simulink model "wlan_phy_rx_pmd"  (Simulink 7.8)
settings: Solver=VariableStepDiscrete  SolverMode=SingleTasking  StartTime=0.0  StopTime=simtime  RelTol=1e-3
PostLoadFcn:
  | wlan_phy_rx_init
InitFcn:
  | wlan_phy_rx_init
StartFcn:
  | wlan_phy_rx_init
values from neighbouring files (symbols this model uses):
  barker_seq20 = 0.95 * circshift(barker_seq20, [0 4]) ./ max(abs(barker_seq20))    [wlan_phy_rx_init.m]
  bit_scrambler_lfsr_bytes = bi2de(reshape(repmat(scr, 1, 8), 8, 127)', 'left-msb')    [wlan_phy_rx_init.m]
  deinterleave_ROM = [deinterleave_ROM interleave_64QAM zeros(1, 512-length(interleave_64QAM))]    [wlan_phy_rx_init.m]
  longCorr_coef_i = [3*real(LTS_corr)]    [wlan_phy_rx_init.m]
  longCorr_coef_q = [3*imag(LTS_corr)]    [wlan_phy_rx_init.m]
  MAX_CP_LEN = 32    [wlan_phy_rx_init.m]
  MAX_NCBPS = 288    [wlan_phy_rx_init.m]
  MAX_NUM_BYTES = 4096    [wlan_phy_rx_init.m]
  MAX_NUM_SC = 64    [wlan_phy_rx_init.m]
  MAX_NUM_SYMS = 600    [wlan_phy_rx_init.m]
  n_bits_preFFT_sampBuff = ceil(log2(4*MAX_NUM_SC))    [wlan_phy_rx_init.m]
  NCBPS_16QAM = 192    [wlan_phy_rx_init.m]
  NCBPS_64QAM = 288    [wlan_phy_rx_init.m]
  NCBPS_BPSK = 48    [wlan_phy_rx_init.m]
  NCBPS_QPSK = 96    [wlan_phy_rx_init.m]
  NDBPS_16QAM12 = 96    [wlan_phy_rx_init.m]
  NDBPS_16QAM34 = 144    [wlan_phy_rx_init.m]
  NDBPS_64QAM23 = 192    [wlan_phy_rx_init.m]
  NDBPS_64QAM34 = 216    [wlan_phy_rx_init.m]
  NDBPS_BPSK12 = 24    [wlan_phy_rx_init.m]
  NDBPS_BPSK34 = 36    [wlan_phy_rx_init.m]
  NDBPS_QPSK12 = 48    [wlan_phy_rx_init.m]
  NDBPS_QPSK34 = 72    [wlan_phy_rx_init.m]
  paylod_vec_samp_time = 8    [wlan_phy_rx_init.m]
  PHY_MIN_PKT_LEN = 14    [wlan_phy_rx_init.m]
  scr_ind_rev = zeros(1,128)    [wlan_phy_rx_init.m]
undefined symbols (not defined in the file or in its folder): gcbh, xlBlockLoadCallback, xlGetNormalizedPeriod, xlipgetpartsetting
libraries: xbsIndex_r4 x3519, simulink x1, xbsEDKLib_r4 x1
(part 4 of 21)

== system wlan_phy_rx_pmd/CFO & Samp Buffer/CFO Correction ==
  note: DDS requires >1 cycle EN early to get setup
      | Use gloabl reset for this
      | Safe, since DDS output is ignored when
      | it's not being used, so bogus outputs
      | between packets is no problem
      |
      | DDS updates once per input sample
      | Since the FFT only consumes 64 samples
      | per OFDM symbol (ignoring the CP),
      | the DDS keeps running after each FFT
      | frame is loaded. This is fine, since the FFT is
      | configured for non-streaming (it ignores its
      | I/Q inputs while computing a transform)
  "I " : Inport
  "Q " : Inport  Port=2
  DDS Run : Inport  Port=3
  Valid : Inport  Port=4
  tlast : Inport  Port=5
  CFO Est : Inport  Port=6
  CFO Corr : Scope  NumInputPorts=8
      settings: TimeRange=35000  YMin=0~-0.4~0~-1~0~0~-0.4~0  YMax=1~0.4~1~2~1~1~0.4~1  SaveName=ScopeData3
  "Complex Multiplier 5.0 " : xbsIndex_r4/Complex Multiplier 5.0   hasatlast=off  hasatuser=on  atuserwidth=1  hasbtlast=off  hasbtuser=off  btuserwidth=1  multtype=Use_Mults  optimizegoal=Performance  flowcontrol=NonBlocking  outputwidth=16  roundmode=Truncate  hasctrltlast=off  hasctrltuser=off  ctrltuserwidth=1  outtlastbehv=Null  latencyconfig=Manual  minimumlatency=2  aclken=off  aresetn=off  trim_axipin_name=on  aportwidth=63  bportwidth=63  ip_name=Complex Multiplier  ip_version=5.0  dsptool_ready=true  ipcore_usecache=true  ipcore_useipmodelcache=true  ipcore_verbose=false  ipcore_latency_parameter='minimumlatency'  wrapper_available=true  port_translation_map={ 'aclken' => 'en', 'aresetn' => 'rst'}  structural_sim=false  block_type=cmpy_v5_0
  Constant1 : xbsIndex_r4/Constant  const=1  gui_display_data_type=Boolean  arith_type=Boolean  n_bits=16  bin_pt=14  preci_type=Single  exp_width=8  frac_width=24  explicit_period=off  period=8  equ=P=C  opselect=C  inp2=PCIN>>17  opr=+  inp1=P  carry=CIN  dbl_ovrd=off  block_type=constant
  Constant2 : xbsIndex_r4/Constant  const=1  gui_display_data_type=Fixed-point  arith_type=Signed (2's comp)  n_bits=16  bin_pt=15  preci_type=Single  exp_width=8  frac_width=24  explicit_period=on  period=1  equ=P=C  opselect=C  inp2=PCIN>>17  opr=+  inp1=P  carry=CIN  dbl_ovrd=off  block_type=constant
  Constant3 : xbsIndex_r4/Constant  const=0  gui_display_data_type=Fixed-point  arith_type=Signed (2's comp)  n_bits=16  bin_pt=15  preci_type=Single  exp_width=8  frac_width=24  explicit_period=on  period=1  equ=P=C  opselect=C  inp2=PCIN>>17  opr=+  inp1=P  carry=CIN  dbl_ovrd=off  block_type=constant
  Constant5 : xbsIndex_r4/Constant  const=1  gui_display_data_type=Boolean  arith_type=Boolean  n_bits=16  bin_pt=14  preci_type=Single  exp_width=8  frac_width=24  explicit_period=off  period=8  equ=P=C  opselect=C  inp2=PCIN>>17  opr=+  inp1=P  carry=CIN  dbl_ovrd=off  block_type=constant
  Convert1 : xbsIndex_r4/Convert  gui_display_data_type=Fixed-point  arith_type="Signed  (2's comp)"  n_bits=16  bin_pt=15  float_type=Single  exp_bits=8  fraction_bits=24  quantization=Truncate  overflow=Wrap  en=off  latency=0  dbl_ovrd=off  pipeline=off  block_type=convert
  Convert2 : xbsIndex_r4/Convert  gui_display_data_type=Fixed-point  arith_type="Signed  (2's comp)"  n_bits=16  bin_pt=15  float_type=Single  exp_bits=8  fraction_bits=24  quantization=Truncate  overflow=Wrap  en=off  latency=0  dbl_ovrd=off  pipeline=off  block_type=convert
  Convert3 : xbsIndex_r4/Convert  gui_display_data_type=Fixed-point  arith_type="Signed  (2's comp)"  n_bits=26  bin_pt=26  float_type=Single  exp_bits=8  fraction_bits=24  quantization=Truncate  overflow=Flag as error  en=off  latency=0  dbl_ovrd=off  pipeline=off  block_type=convert
  Convert4 : xbsIndex_r4/Convert  gui_display_data_type=Fixed-point  arith_type=Unsigned  n_bits=1  bin_pt=0  float_type=Single  exp_bits=8  fraction_bits=24  quantization=Truncate  overflow=Flag as error  en=off  latency=0  dbl_ovrd=off  pipeline=off  block_type=convert
  Convert5 : xbsIndex_r4/Convert  gui_display_data_type=Boolean  arith_type=Boolean  n_bits=1  bin_pt=0  float_type=Single  exp_bits=8  fraction_bits=24  quantization=Truncate  overflow=Flag as error  en=off  latency=0  dbl_ovrd=off  pipeline=off  block_type=convert
  DDS Compiler 4.0 : xbsIndex_r4/DDS Compiler 4.0   partspresent=Phase_Generator_and_SIN_COS_LUT  dds_clock_rate=160  channels=1  parameter_entry=Hardware_Parameters  spurious_free_dynamic_range=36  frequency_resolution=0.4  noise_shaping=None  phase_width=26  output_width=16  output_selection=Sine_and_Cosine  negative_sine=off  negative_cosine=off  amplitude_mode=Full_Range  memory_type=Auto  optimization_goal=Auto  dsp48_use=Minimal  latency_configuration=Auto  latency=6  has_phase_out=off  sclr_pin=off  clock_enable=on  rfd=off  rdy=on  channel_pin=off  explicit_period=off  period=1  phase_increment=Programmable  output_frequency1=0  output_frequency2=0  output_frequency3=0  output_frequency4=0  output_frequency5=0  output_frequency6=0  output_frequency7=0  output_frequency8=0  output_frequency9=0  output_frequency10=0  output_frequency11=0  output_frequency12=0  output_frequency13=0  output_frequency14=0  output_frequency15=0  output_frequency16=0  pinc1='0'  pinc2='0'  pinc3='0'  pinc4='0'  pinc5='0'  pinc6='0'  pinc7='0'  pinc8='0'  pinc9='0'  pinc10='0'  pinc11='0'  pinc12='0'  pinc13='0'  pinc14='0'  pinc15='0'  pinc16='0'  phase_offset=None  phase_offset_angles1=0  phase_offset_angles2=0  phase_offset_angles3=0  phase_offset_angles4=0  phase_offset_angles5=0  phase_offset_angles6=0  phase_offset_angles7=0  phase_offset_angles8=0  phase_offset_angles9=0  phase_offset_angles10=0  phase_offset_angles11=0  phase_offset_angles12=0  phase_offset_angles13=0  phase_offset_angles14=0  phase_offset_angles15=0  phase_offset_angles16=0  poff1='0'  poff2='0'  poff3='0'  poff4='0'  poff5='0'  poff6='0'  poff7='0'  poff8='0'  poff9='0'  poff10='0'  poff11='0'  poff12='0'  poff13='0'  poff14='0'  poff15='0'  poff16='0'  por_mode=false  gui_behaviour=Sysgen  ip_name=DDS Compiler  ip_version=4.0  dsptool_ready=true  wrapper_available=true  port_translation_map={ 'ce' => 'en', 'sclr' => 'rst' }  ipcore_xco_need_fpga_part=true  ipcore_fpga_part=xlipgetpartsetting(gcb, {'virtex4', 'xc4vsx35', '-10', 'ff668'})  ipcore_usecache=true  ipcore_useipmodelcache=true  ipcore_verbose=false  block_type=dds_compiler_v4_0
  Gateway Out{1..7,17} (8 alike) : xbsIndex_r4/Gateway Out  inherit_from_input=off  hdl_port=off  timing_constraint=None  locs_specified=off  LOCs={}  UseAsDAC=off  DACChannel='1'  block_type=gatewayout
  Logical2 : xbsIndex_r4/Logical  logical_function=OR  inputs=3  en=off  latency=0  precision=Full  arith_type=Unsigned  n_bits=16  bin_pt=0  align_bp=on  dbl_ovrd=off  block_type=logical
  Sim Reset : Subsystem
  valid : Outport
  I : Outport  Port=2
  Q : Outport  Port=3
  " tlast" : Outport  Port=4
  wires:
  Constant1/1 -> DDS Compiler 4.0/1
  CFO Est/1 -> Convert3/1
  DDS Run/1 -> Logical2/1
  "Complex Multiplier 5.0 "/2 -> Convert2/1, Gateway Out6/1
  "Complex Multiplier 5.0 "/3 -> Convert1/1
  "I "/1 -> "Complex Multiplier 5.0 "/3, Gateway Out1/1
  "Q "/1 -> "Complex Multiplier 5.0 "/2
  Gateway Out17/1 -> CFO Corr/1
  Gateway Out1/1 -> CFO Corr/2
  Gateway Out2/1 -> CFO Corr/3
  Gateway Out3/1 -> CFO Corr/4
  Gateway Out4/1 -> CFO Corr/5
  DDS Compiler 4.0/2 -> "Complex Multiplier 5.0 "/6, Gateway Out3/1
  Valid/1 -> "Complex Multiplier 5.0 "/1, Gateway Out2/1
  Convert1/1 -> I/1
  Convert2/1 -> Q/1
  "Complex Multiplier 5.0 "/1 -> valid/1, Gateway Out5/1
  DDS Compiler 4.0/3 -> "Complex Multiplier 5.0 "/7
  Logical2/1 -> DDS Compiler 4.0/3, Gateway Out17/1
  [RX_GLOBAL_RESET] -> Logical2/2
  Convert3/1 -> DDS Compiler 4.0/2
  Constant5/1 -> "Complex Multiplier 5.0 "/5
  tlast/1 -> Convert4/1
  "Complex Multiplier 5.0 "/4 -> Gateway Out7/1, Convert5/1
  Gateway Out5/1 -> CFO Corr/6
  Gateway Out6/1 -> CFO Corr/7
  Gateway Out7/1 -> CFO Corr/8
  Convert4/1 -> "Complex Multiplier 5.0 "/4, Gateway Out4/1
  Convert5/1 -> " tlast"/1
  Sim Reset/1(Out1) -> Logical2/3

== system wlan_phy_rx_pmd/CFO & Samp Buffer/CFO Estimation ==
  note: (1/2) for normalization of phase
      | (1/64) for number of summed elements
  LTS_Sync : Inport
  rx_I : Inport  Port=2
  rx_Q : Inport  Port=3
  rx_valid : Inport  Port=4
  CFO Est : Scope  NumInputPorts=10
      settings: TimeRange=10000  YMin=-1~-1~-0.25~-1~-10~-2.5~-1~0~0~-1  YMax=1~1~2~2~50~15~1~1~0.001~1
  Conj Mult : Subsystem
  Convert2 : xbsIndex_r4/Convert  gui_display_data_type=Fixed-point  arith_type="Signed  (2's comp)"  n_bits=21  bin_pt=13  float_type=Single  exp_bits=8  fraction_bits=24  quantization=Truncate  overflow=Flag as error  en=off  latency=0  dbl_ovrd=off  pipeline=off  block_type=convert
  Delay : xbsIndex_r4/Delay  rst=off  infoeditControl=Selection of Reset will increase slice count due to use of real FFs and instead of SRLs  en=off  latency=8  dbl_ovrd=off  reg_retiming=off  block_type=delay
  Delay1 : xbsIndex_r4/Delay  rst=off  infoeditControl=Selection of Reset will increase slice count due to use of real FFs and instead of SRLs  en=on  latency=64  dbl_ovrd=off  reg_retiming=off  block_type=delay
      settings: xl_area=[7 14 0 14 0 0 0]  block_version=VER_STRING_GOES_HERE
  Delay2 : xbsIndex_r4/Delay  rst=off  infoeditControl=Selection of Reset will increase slice count due to use of real FFs and instead of SRLs  en=on  latency=64  dbl_ovrd=off  reg_retiming=off  block_type=delay
      settings: xl_area=[7 14 0 14 0 0 0]  block_version=VER_STRING_GOES_HERE
  Delay3 : xbsIndex_r4/Delay  rst=off  infoeditControl=Selection of Reset will increase slice count due to use of real FFs and instead of SRLs  en=on  latency=60  dbl_ovrd=off  reg_retiming=off  block_type=delay
      settings: xl_area=[7 14 0 14 0 0 0]  block_version=VER_STRING_GOES_HERE
  Gateway Out{,1..8} (9 alike) : xbsIndex_r4/Gateway Out  inherit_from_input=off  hdl_port=off  timing_constraint=None  locs_specified=off  LOCs={}  UseAsDAC=off  DACChannel='1'  block_type=gatewayout
  Phase : Subsystem
  Posedge3 : Subsystem
  Register : xbsIndex_r4/Register  init=0  rst=on  en=on  dbl_ovrd=off  block_type=register
  Running Sum : Subsystem
  Running Sum1 : Subsystem
  S-R Latch : Subsystem
  Scale : xbsIndex_r4/Scale  scale_factor=-7  dbl_ovrd=off  block_type=scale
      settings: block_version=10.1.2
  CFO : Outport
  wires:
  Gateway Out5/1 -> CFO Est/6  "Sum Q"
  Gateway Out4/1 -> CFO Est/5  "Sum I"
  Gateway Out3/1 -> CFO Est/4  "Conj Prod Q"
  Gateway Out2/1 -> CFO Est/3  "Conj Prod I"
  Gateway Out1/1 -> CFO Est/2  "ADC Q"
  Gateway Out/1 -> CFO Est/1  "ADC I"
  rx_valid/1 -> Conj Mult/1(Valid), Delay2/2, Delay1/2, Delay3/2
  rx_I/1 -> Conj Mult/2(A I), Delay2/1, Gateway Out/1
  rx_Q/1 -> Conj Mult/3(A Q), Delay1/1, Gateway Out1/1
  Delay2/1 -> Conj Mult/4(B I)
  Delay1/1 -> Conj Mult/5(B Q)
  Conj Mult/2(" I") -> Running Sum/2(D), Gateway Out2/1
  Conj Mult/1(" Valid") -> Running Sum/1(En), Running Sum1/1(En)
  Conj Mult/3(" Q") -> Running Sum1/2(D), Gateway Out3/1
  Running Sum/1(Valid) -> Phase/1(Valid)
  Phase/2(Phase) -> Gateway Out6/1, Register/1
  Gateway Out6/1 -> CFO Est/7  "Phase(Sum)"
  Register/1 -> Convert2/1
  Convert2/1 -> Scale/1
  Scale/1 -> CFO/1, Gateway Out8/1
  Gateway Out7/1 -> CFO Est/8  "LTS Sync"
  LTS_Sync/1 -> S-R Latch/1(S)
  Gateway Out8/1 -> CFO Est/9  "CFO Est Out"
  ? -> CFO Est/10
  Delay3/1 -> Posedge3/1(D)
  S-R Latch/1(Q) -> Delay/1, Delay3/1
  Delay/1 -> S-R Latch/2(R)
  Posedge3/1(Q) -> Gateway Out7/1, Register/3
  Running Sum/2(Sum) -> Phase/2(Re), Gateway Out4/1
  Running Sum1/2(Sum) -> Phase/3(Im), Gateway Out5/1
  [regRx_BYPASS_CFO_EST] -> Register/2

== system wlan_phy_rx_pmd/CFO & Samp Buffer/FIFO ==
  note: AND not strictly necessary, since FFT
      | only honors tlast when tvalid is asserted.
      | Explicit AND here makes scopes
      | much easier to understand.
  note: This FIFO aids in synchronizing sample generation
      | and feeding the FFT. It would be possible to design
      | the sample buffer control logic not to require
      | this FIFO, but then the logic would need tweaking
      | if the FFT timing or sample processing timing
      | ever change. This FIFO isolates those blocks,
      | at the cost of a little memory and 1 clock cyle latency
      | (assuming the FFT consumes samples faster than
      | they're received)
  data tvalid : Inport
  I : Inport  Port=2
  Q : Inport  Port=3
  data tlast : Inport  Port=4
  FFT tready : Inport  Port=5
  reset : Inport  Port=6
  AXI FIFO : xbsIndex_r4/AXI FIFO  input_depth_axis=128  actual_fifo_depth_label=130  enable_tdata=on  enable_tdest=off  enable_tstrb=off  enable_tready=on  enable_tid=off  enable_tuser=off  enable_tkeep=off  enable_tlast=on  has_aresetn=off  enable_data_counts_axis=on  fifo_implementation_type_axis=Common Clock Block RAM  trim_axipin_name=on  programmable_full_type_axis=Full  full_threshold_assert_value_axis=0  programmable_empty_type_axis=Empty  empty_threshold_assert_value_axis=1022  block_type=axi_fifo
  FIFO : Scope  NumInputPorts=9
      settings: TimeRange=14580  YMin=0.95~-1~-1~-1~0.95~-1~-1~-1~-1  YMax=1.05~1~1~1~1.05~1~1~1~1  SaveName=ScopeData20
  Gateway Out{1..6,8..9} (8 alike) : xbsIndex_r4/Gateway Out  inherit_from_input=off  hdl_port=off  timing_constraint=None  locs_specified=off  LOCs={}  UseAsDAC=off  DACChannel='1'  block_type=gatewayout
  I/Q Concat : Subsystem
  I/Q Slice : Subsystem
  Inverter : xbsIndex_r4/Inverter  en=off  latency=0  dbl_ovrd=off  block_type=inv
  Logical2 : xbsIndex_r4/Logical  logical_function=AND  inputs=2  en=off  latency=0  precision=Full  arith_type=Unsigned  n_bits=16  bin_pt=0  align_bp=on  dbl_ovrd=off  block_type=logical
  Register2 : xbsIndex_r4/Register  init=1  rst=off  en=off  dbl_ovrd=off  block_type=register
      settings: xl_area=[1 1 0 0 0 0 0]  block_version=VER_STRING_GOES_HERE
  FFT tvalid : Outport
  FFT tdata_re : Outport  Port=2
  FFT tdata_im : Outport  Port=3
  FFT tlast : Outport  Port=4
  FIFO tready : Outport  Port=5
  wires:
  AXI FIFO/2 -> I/Q Slice/1(IQ)
  AXI FIFO/1 -> FFT tvalid/1, Gateway Out1/1, Logical2/1
  AXI FIFO/3 -> Logical2/2
  Gateway Out8/1 -> FIFO/1  "FFT ready"
  I/Q Slice/1(I) -> FFT tdata_re/1
  I/Q Slice/2(Q) -> FFT tdata_im/1, Gateway Out2/1
  FFT tready/1 -> Gateway Out8/1, AXI FIFO/1
  data tvalid/1 -> Gateway Out5/1, AXI FIFO/2
  data tlast/1 -> Gateway Out6/1, AXI FIFO/4
  AXI FIFO/4 -> FIFO tready/1, Gateway Out4/1
  Gateway Out1/1 -> FIFO/2  "FIFO out valid"
  Gateway Out2/1 -> FIFO/3  "Q out"
  Gateway Out3/1 -> FIFO/4  "tlast out"
  Gateway Out4/1 -> FIFO/5  "FIFO ready"
  Gateway Out5/1 -> FIFO/6  "valid in"
  Gateway Out6/1 -> FIFO/7  "tlast in"
  Logical2/1 -> FFT tlast/1, Gateway Out3/1
  I/1 -> I/Q Concat/1(I)
  Q/1 -> I/Q Concat/2(Q)
  I/Q Concat/1(IQ) -> AXI FIFO/3
  reset/1 -> Inverter/1
  Inverter/1 -> Register2/1
  ? -> FIFO/8  "Reset"
  Gateway Out9/1 -> FIFO/9  "FIFO Occ"
  AXI FIFO/5 -> Gateway Out9/1
  Register2/1 -> ?

== system wlan_phy_rx_pmd/CFO & Samp Buffer/Negedge ==
  D : Inport
  Delay : xbsIndex_r4/Delay  rst=off  infoeditControl=Selection of Reset will increase slice count due to use of real FFs and instead of SRLs  en=off  latency=1  dbl_ovrd=off  reg_retiming=off  block_type=delay
      settings: infoedit=Hardware notes: A delay line is a chain, each link of which is an SRL16 followed by a flip-flop. If register retiming is enabled, the delay line is a chain of flip-flops.  xl_area=[1 1 0 0 0 0 0]  block_version=VER_STRING_GOES_HERE
  Inverter : xbsIndex_r4/Inverter  en=off  latency=0  dbl_ovrd=off  block_type=inv
      settings: xl_area=[0 0 0 0 0 0 0]  block_version=VER_STRING_GOES_HERE
  Logical1 : xbsIndex_r4/Logical  logical_function=AND  inputs=2  en=off  latency=0  precision=Full  arith_type=Unsigned  n_bits=8  bin_pt=2  align_bp=on  dbl_ovrd=off  block_type=logical
      settings: xl_area=[1 0 0 1 0 0 0]  block_version=VER_STRING_GOES_HERE
  Q : Outport
  wires:
  D/1 -> Delay/1, Inverter/1
  Logical1/1 -> Q/1
  Delay/1 -> Logical1/1
  Inverter/1 -> Logical1/2

== system wlan_phy_rx_pmd/CFO & Samp Buffer/Samp Buffer ==
  note: Circular sample buffer records all incoming I/Q samples post pkt det
      | Samples are unloaded from the buffer after LTS sync and fed into
      | the FFT (via CFO correction and a FIFO)
      |
      | This buffer is required because 802.11 uses the same preamble samples
      | (the LTS) for sample-level synchronization and CFO estimation. The
      | received LTS samples must be saved and CFO-corrected before the
      | FFT to avoid degrading the channel estimates.
  Pkt Det : Inport
  Pkt Done : Inport  Port=2
  rx I : Inport  Port=3
  rx Q : Inport  Port=4
  IQ valid : Inport  Port=5
  LTS Sync 2 : Inport  Port=6
  FIFO tready : Inport  Port=7
  Circular Sample Buffer : xbsIndex_r4/Dual Port RAM  depth=2^n_bits_preFFT_sampBuff  initVector=1 * repmat([0:63], 1, 4)  distributed_mem=Block RAM  init_a=0  init_b=0  rst_a=off  rst_b=off  en_a=off  en_b=off  latency=1  write_mode_A=Read After Write  write_mode_B=Read After Write  dbl_ovrd=off  optimize=Area  block_type=dpram
  Concat : Subsystem
  Constant3 : xbsIndex_r4/Constant  const=0  gui_display_data_type=Fixed-point  arith_type=Unsigned  n_bits=32  bin_pt=0  preci_type=Single  exp_width=8  frac_width=24  explicit_period=off  period=8  equ=P=C  opselect=C  inp2=PCIN>>17  opr=+  inp1=P  carry=CIN  dbl_ovrd=off  block_type=constant
  Constant4 : xbsIndex_r4/Constant  const=0  gui_display_data_type=Boolean  arith_type=Boolean  n_bits=6  bin_pt=0  preci_type=Single  exp_width=8  frac_width=24  explicit_period=off  period=8  equ=P=C  opselect=C  inp2=PCIN>>17  opr=+  inp1=P  carry=CIN  dbl_ovrd=off  block_type=constant
  Counter : xbsIndex_r4/Counter  cnt_type=Free Running  cnt_to=Inf  operation=Up  start_count=0  cnt_by_val=1  arith_type=Unsigned  n_bits=n_bits_preFFT_sampBuff  bin_pt=0  load_pin=off  rst=off  en=on  explicit_period=off  period=8  dbl_ovrd=off  use_behavioral_HDL=off  implementation=Fabric  block_type=counter
  Delay : xbsIndex_r4/Delay  rst=off  infoeditControl=Selection of Reset will increase slice count due to use of real FFs and instead of SRLs  en=off  latency=1  dbl_ovrd=off  reg_retiming=off  block_type=delay
  Delay2 : xbsIndex_r4/Delay  rst=off  infoeditControl=Selection of Reset will increase slice count due to use of real FFs and instead of SRLs  en=off  latency=1  dbl_ovrd=off  reg_retiming=off  block_type=delay
  FFT Load Ctrl : Subsystem
  FSM : Subsystem
  Gateway Out{1..7} (7 alike) : xbsIndex_r4/Gateway Out  inherit_from_input=off  hdl_port=off  timing_constraint=None  locs_specified=off  LOCs={}  UseAsDAC=off  DACChannel='1'  block_type=gatewayout
  Logical2 : xbsIndex_r4/Logical  logical_function=AND  inputs=2  en=off  latency=0  precision=Full  arith_type=Unsigned  n_bits=16  bin_pt=0  align_bp=on  dbl_ovrd=off  block_type=logical
  Posedge : Subsystem
  Posedge3 : Subsystem
  Register : xbsIndex_r4/Register  init=0  rst=off  en=off  dbl_ovrd=off  block_type=register
  Register1 : xbsIndex_r4/Register  init=0  rst=off  en=off  dbl_ovrd=off  block_type=register
  S-R Latch : Subsystem
  Scope : Scope  NumInputPorts=7
      settings: TimeRange=10000  YMin=-0.25~-0.25~0~0~0~-0.1~-0.1  YMax=0.2~0.2~300~300~4000~1.1~1.1  SaveName=ScopeData25
  Slice : Subsystem
  Terminator : Terminator
  dbg_payload : xbsIndex_r4/Gateway Out  inherit_from_input=off  hdl_port=on  timing_constraint=None  locs_specified=off  LOCs={}  UseAsDAC=off  DACChannel='1'  block_type=gatewayout
  I : Outport
  Q : Outport  Port=2
  Buff Rd : Outport  Port=3
  " IQ valid" : Outport  Port=4
  tlast : Outport  Port=5
  tag scope: OFDM_RX_RUNNING_DSSS_BLOCK=global
  wires:
  Concat/1(IQ) -> Circular Sample Buffer/2
  Circular Sample Buffer/1 -> Terminator/1
  FFT Load Ctrl/1(Rd Addr) -> Circular Sample Buffer/4, Gateway Out1/1
  Constant3/1 -> Circular Sample Buffer/5
  Constant4/1 -> Circular Sample Buffer/6
  Logical2/1 -> Posedge/1(D)
  Posedge/1(Q) -> FFT Load Ctrl/3(LTS Sync), FSM/2(LTS)
  FFT Load Ctrl/5(FFT Load Done) -> FSM/1(FFT Load Done), Gateway Out7/1
  FSM/2(FFT Data load) -> FFT Load Ctrl/5(FFT Load)
  Circular Sample Buffer/2 -> Slice/1(IQ)
  FSM/1(FFT skip sel) -> FFT Load Ctrl/4(Skip Sel)
  FFT Load Ctrl/3(FFT tvalid) -> Delay/1, Posedge3/1(D)
  FFT Load Ctrl/2(Reading) -> Delay2/1
  Pkt Done/1 -> FSM/3(Pkt Done), S-R Latch/2(R), Gateway Out4/1
  rx I/1 -> Concat/1(I), Gateway Out6/1
  rx Q/1 -> Concat/2(Q)
  IQ valid/1 -> Circular Sample Buffer/3, Counter/1
  Slice/1(I) -> I/1, Gateway Out5/1
  Slice/2(Q) -> Q/1
  FIFO tready/1 -> FFT Load Ctrl/2(data tready)
  Delay2/1 -> Buff Rd/1
  Delay/1 -> " IQ valid"/1
  FFT Load Ctrl/4(FFT tlast) -> tlast/1
  LTS Sync 2/1 -> Logical2/1
  Pkt Det/1 -> Logical2/2, Gateway Out3/1
  Gateway Out5/1 -> Scope/2  "FFT In I"
  Gateway Out1/1 -> Scope/3  "Rd Addr"
  Gateway Out2/1 -> Scope/4  "Wr Addr"
  Gateway Out3/1 -> Scope/5  "Pkt Det"
  Posedge3/1(Q) -> S-R Latch/1(S)
  S-R Latch/1(Q) -> Register/1, [OFDM_RX_RUNNING_DSSS_BLOCK]
  Gateway Out4/1 -> Scope/6  "Pkt Done"
  Gateway Out6/1 -> Scope/1  "ADC I"
  Register/1 -> Register1/1
  Register1/1 -> dbg_payload/1
  Gateway Out7/1 -> Scope/7  "FFT Load Done"
  Counter/1 -> Circular Sample Buffer/1, FFT Load Ctrl/1(Wr Addr), Gateway Out2/1
  FSM/3(FFT LTS load) -> FFT Load Ctrl/6(FFT LTS Load)

== system wlan_phy_rx_pmd/Chan Est & EQ/Chan Est ==
  note: Scale by 2^-1, since est values
      | are summed from 2 training syms
  x_re : Inport
  x_im : Inport  Port=2
  x_ind : Inport  Port=3
  x_valid : Inport  Port=4
  Sym Ind : Inport  Port=5
  Abs : Abs
  Abs1 : Abs
  Chan Est Offload : Subsystem
  Constant1 : xbsIndex_r4/Constant  const=2  gui_display_data_type=Fixed-point  arith_type=Unsigned  n_bits=2  bin_pt=0  preci_type=Single  exp_width=8  frac_width=24  explicit_period=off  period=8  equ=P=C  opselect=C  inp2=PCIN>>17  opr=+  inp1=P  carry=CIN  dbl_ovrd=off  block_type=constant
  Constant2 : xbsIndex_r4/Constant  const=0  gui_display_data_type=Fixed-point  arith_type=Unsigned  n_bits=2  bin_pt=0  preci_type=Single  exp_width=8  frac_width=24  explicit_period=off  period=8  equ=P=C  opselect=C  inp2=PCIN>>17  opr=+  inp1=P  carry=CIN  dbl_ovrd=off  block_type=constant
  Delay{1..4,7} (5 alike) : xbsIndex_r4/Delay  rst=off  infoeditControl=Selection of Reset will increase slice count due to use of real FFs and instead of SRLs  en=off  latency=2  dbl_ovrd=off  reg_retiming=off  block_type=delay
      settings: xl_area=[0, 0, 0, 0, 0, 0, 0]  block_version=VER_STRING_GOES_HERE
  Gateway Out{1..5,8} (6 alike) : xbsIndex_r4/Gateway Out  inherit_from_input=off  hdl_port=off  timing_constraint=None  locs_specified=off  LOCs={}  UseAsDAC=off  DACChannel='1'  block_type=gatewayout
  H Est Outputs : Scope  NumInputPorts=8
      settings: TimeRange=4000  YMin=-0.5~-1~-0.6000000000000001~-0.5~0~0~0~0  YMax=0.5~0.5~0.3999999999999999~1~80~1~3~0.8  SaveName=ScopeData16
  I Estimate Buffer : Subsystem
  Inverter : xbsIndex_r4/Inverter  en=off  latency=0  dbl_ovrd=off  block_type=inv
  Logical1 : xbsIndex_r4/Logical  logical_function=AND  inputs=2  en=off  latency=0  precision=Full  arith_type=Unsigned  n_bits=16  bin_pt=0  align_bp=on  dbl_ovrd=off  block_type=logical
  Logical2 : xbsIndex_r4/Logical  logical_function=AND  inputs=2  en=off  latency=0  precision=Full  arith_type=Unsigned  n_bits=16  bin_pt=0  align_bp=on  dbl_ovrd=off  block_type=logical
  Mult by LTS : Subsystem
  Q Estimate Buffer : Subsystem
  Real-Imag to Complex : RealImagToComplex
  Real-Imag to Complex1 : RealImagToComplex
  Relational1 : xbsIndex_r4/Relational  mode=a<b  en=off  latency=0  dbl_ovrd=off  block_type=relational
  Relational2 : xbsIndex_r4/Relational  mode=a=b  en=off  latency=0  dbl_ovrd=off  block_type=relational
  Scale : xbsIndex_r4/Scale  scale_factor=-1  dbl_ovrd=off  block_type=scale
  Scale1 : xbsIndex_r4/Scale  scale_factor=-1  dbl_ovrd=off  block_type=scale
  To Float : Subsystem
  " x_I" : Outport
  " x_Q" : Outport  Port=2
  H_I : Outport  Port=3
  H_Q : Outport  Port=4
  " valid" : Outport  Port=5
  " x_ind" : Outport  Port=6
  " sym_ind" : Outport  Port=7
  wires:
  Logical2/1 -> I Estimate Buffer/3(Est_WE), Q Estimate Buffer/3(Est_WE)
  Constant2/1 -> Relational2/1
  x_valid/1 -> Logical2/1, Logical1/1
  Sym Ind/1 -> Relational2/2, Relational1/1, Delay2/1
  Constant1/1 -> Relational1/2
  Relational2/1 -> I Estimate Buffer/4(First Sym), Q Estimate Buffer/4(First Sym)
  Relational1/1 -> Inverter/1, Logical2/2
  Delay7/1 -> Gateway Out4/1, To Float/5(x_ind), Chan Est Offload/1(x_ind)
  Delay1/1 -> Gateway Out5/1, To Float/6(x_valid), Chan Est Offload/4(x_valid)
  Logical1/1 -> Delay1/1
  Inverter/1 -> Logical1/2
  I Estimate Buffer/1(h[n]) -> Scale/1
  Q Estimate Buffer/1(h[n]) -> Scale1/1
  Delay2/1 -> To Float/7(sym_ind), Chan Est Offload/5(sym_ind)
  Delay3/1 -> Gateway Out8/1, To Float/1("x_I ")
  Delay4/1 -> Gateway Out1/1, To Float/2("x_Q ")
  Gateway Out8/1 -> H Est Outputs/1, Real-Imag to Complex/1  "x_I"
  Gateway Out1/1 -> H Est Outputs/2, Real-Imag to Complex/2  "x_Q"
  Gateway Out2/1 -> H Est Outputs/3, Real-Imag to Complex1/1  "H_I"
  Gateway Out3/1 -> H Est Outputs/4, Real-Imag to Complex1/2  "H_Q"
  Gateway Out4/1 -> H Est Outputs/5  "SC Ind"
  Gateway Out5/1 -> H Est Outputs/6  "Valid Out"
  x_ind/1 -> Delay7/1, Q Estimate Buffer/2(Est_Addr), I Estimate Buffer/2(Est_Addr), Mult by LTS/3(x_ind)
  x_re/1 -> Delay3/1, Mult by LTS/1(x_re)
  x_im/1 -> Delay4/1, Mult by LTS/2(x_im)
  Mult by LTS/2(H_im) -> Q Estimate Buffer/1(Est_Data)
  Mult by LTS/1(H_re) -> I Estimate Buffer/1(Est_Data)
  Scale/1 -> Gateway Out2/1, To Float/3("H_I "), Chan Est Offload/2(x_I)
  Scale1/1 -> Gateway Out3/1, To Float/4("H_Q "), Chan Est Offload/3(x_Q)
  To Float/1(" x_I") -> " x_I"/1
  To Float/2(" x_Q") -> " x_Q"/1
  To Float/3(H_I) -> H_I/1
  To Float/4(" H_Q") -> H_Q/1
  To Float/5(" x_ind") -> " x_ind"/1
  To Float/6(" x_valid") -> " valid"/1
  To Float/7(" sym_ind") -> " sym_ind"/1
  Abs/1 -> H Est Outputs/8
  Real-Imag to Complex/1 -> Abs/1
  Real-Imag to Complex1/1 -> Abs1/1
  Abs1/1 -> H Est Outputs/7

== system wlan_phy_rx_pmd/Chan Est & EQ/EQ & Pilot Processing ==
  note: Delay here so phase error is ready just in time for
      | post-EQ symbols at Phase Correction CORDIC
  x_I : Inport
  x_Q : Inport  Port=2
  H_I : Inport  Port=3
  H_Q : Inport  Port=4
  x_valid : Inport  Port=5
  x_ind : Inport  Port=6
  Sym ind : Inport  Port=7
  Convert : xbsIndex_r4/Convert  gui_display_data_type=Fixed-point  arith_type="Signed  (2's comp)"  n_bits=16  bin_pt=14  float_type=Single  exp_bits=8  fraction_bits=24  quantization="Round  (unbiased: +/- Inf)"  overflow=Saturate  en=off  latency=1  dbl_ovrd=off  pipeline=off  block_type=convert
  Convert1 : xbsIndex_r4/Convert  gui_display_data_type=Fixed-point  arith_type="Signed  (2's comp)"  n_bits=16  bin_pt=14  float_type=Single  exp_bits=8  fraction_bits=24  quantization="Round  (unbiased: +/- Inf)"  overflow=Saturate  en=off  latency=1  dbl_ovrd=off  pipeline=off  block_type=convert
  Debug Outputs : Subsystem
  Delay{1,3..5,8..9} (6 alike) : xbsIndex_r4/Delay  rst=off  infoeditControl=Selection of Reset will increase slice count due to use of real FFs and instead of SRLs  en=off  latency=62  dbl_ovrd=off  reg_retiming=off  block_type=delay
  Delay12 : xbsIndex_r4/Delay  rst=off  infoeditControl=Selection of Reset will increase slice count due to use of real FFs and instead of SRLs  en=off  latency=23  dbl_ovrd=off  reg_retiming=off  block_type=delay
  Delay14 : xbsIndex_r4/Delay  rst=off  infoeditControl=Selection of Reset will increase slice count due to use of real FFs and instead of SRLs  en=off  latency=23  dbl_ovrd=off  reg_retiming=off  block_type=delay
  Delay15 : xbsIndex_r4/Delay  rst=off  infoeditControl=Selection of Reset will increase slice count due to use of real FFs and instead of SRLs  en=off  latency=24  dbl_ovrd=off  reg_retiming=off  block_type=delay
  Delay17 : xbsIndex_r4/Delay  rst=off  infoeditControl=Selection of Reset will increase slice count due to use of real FFs and instead of SRLs  en=off  latency=23  dbl_ovrd=off  reg_retiming=off  block_type=delay
  Delay18 : xbsIndex_r4/Delay  rst=off  infoeditControl=Selection of Reset will increase slice count due to use of real FFs and instead of SRLs  en=off  latency=24  dbl_ovrd=off  reg_retiming=off  block_type=delay
  Delay2 : xbsIndex_r4/Delay  rst=off  infoeditControl=Selection of Reset will increase slice count due to use of real FFs and instead of SRLs  en=off  latency=8  dbl_ovrd=off  reg_retiming=off  block_type=delay
  Delay6 : xbsIndex_r4/Delay  rst=off  infoeditControl=Selection of Reset will increase slice count due to use of real FFs and instead of SRLs  en=off  latency=8  dbl_ovrd=off  reg_retiming=off  block_type=delay
  Delay7 : xbsIndex_r4/Delay  rst=off  infoeditControl=Selection of Reset will increase slice count due to use of real FFs and instead of SRLs  en=off  latency=8  dbl_ovrd=off  reg_retiming=off  block_type=delay
  Divide : xbsIndex_r4/Divide  flow_control=NonBlocking  axi_optimize_goal=Resources  algorithm_type=High_Radix  infoedit1=Specify output fraction-width for Fixed-point division.  fractional_width=16  latency=21  rate=1  has_a_tlast=off  has_a_tuser=off  has_b_tlast=off  has_b_tuser=off  en=off  has_result_tready=off  result_tlast_behv=Pass_A_TLAST  has_underflow=off  has_overflow=off  has_invalid_op=off  has_divide_by_zero=off  fpo_op_type=mergeddivide  block_type=divide
      settings: infoedit=Blocking: Wait for data on all inputs, allow back-pressure.<br>NonBlocking: operate every cycle in which all inputs are valid, no back-pressure.
  Divide1 : xbsIndex_r4/Divide  flow_control=NonBlocking  axi_optimize_goal=Resources  algorithm_type=High_Radix  infoedit1=Specify output fraction-width for Fixed-point division.  fractional_width=16  latency=21  rate=1  has_a_tlast=off  has_a_tuser=off  has_b_tlast=off  has_b_tuser=off  en=off  has_result_tready=off  result_tlast_behv=Pass_A_TLAST  has_underflow=off  has_overflow=off  has_invalid_op=off  has_divide_by_zero=off  fpo_op_type=mergeddivide  block_type=divide
      settings: infoedit=Blocking: Wait for data on all inputs, allow back-pressure.<br>NonBlocking: operate every cycle in which all inputs are valid, no back-pressure.
  EQ : Scope  NumInputPorts=8
      settings: TimeRange=6000  YMin=-1~-0.5~0~0~0~-2~-1~0  YMax=1~1~1~100~3~2~2~1  SaveName=ScopeData9
  Gateway Out{,1..7} (8 alike) : xbsIndex_r4/Gateway Out  inherit_from_input=off  hdl_port=off  timing_constraint=None  locs_specified=off  LOCs={}  UseAsDAC=off  DACChannel='1'  block_type=gatewayout
  Mag : Subsystem
  Phase Correction : Subsystem
  Phase Err Calc : Subsystem
  Register3 : xbsIndex_r4/Register  init=0  rst=off  en=off  dbl_ovrd=off  block_type=register
  Register4 : xbsIndex_r4/Register  init=0  rst=off  en=off  dbl_ovrd=off  block_type=register
  Register5 : xbsIndex_r4/Register  init=0  rst=off  en=off  dbl_ovrd=off  block_type=register
  a*conj(b) : Subsystem
  " x_I" : Outport
  " x_Q" : Outport  Port=2
  " x_ind" : Outport  Port=3
  " x_valid" : Outport  Port=4
  " sym ind" : Outport  Port=5
  wires:
  x_ind/1 -> Gateway Out5/1, Delay2/1
  Sym ind/1 -> Gateway Out6/1, Delay7/1
  x_I/1 -> a*conj(b)/1(A_I), Gateway Out/1
  x_Q/1 -> a*conj(b)/2(A_Q), Gateway Out1/1
  H_I/1 -> a*conj(b)/3(B_I), Mag/1(I)
  H_Q/1 -> a*conj(b)/4(B_Q), Mag/2(Q)
  x_valid/1 -> Gateway Out4/1, Delay6/1
  Delay1/1 -> Register4/1
  Delay3/1 -> Register3/1
  Phase Err Calc/1(Sym Phase Err) -> Phase Correction/3(Phase)
  Delay7/1 -> Phase Err Calc/5(sym_ind), Delay9/1
  a*conj(b)/1(P_I) -> Phase Err Calc/1(CP_I), Delay1/1
  a*conj(b)/2(P_Q) -> Phase Err Calc/2(CP_Q), Delay3/1
  Delay2/1 -> Phase Err Calc/4(x_ind), Delay5/1
  Mag/1(M) -> Delay8/1
  Delay8/1 -> Register5/1
  Divide/1 -> Convert/1
  Divide1/1 -> Convert1/1
  Convert/1 -> Phase Correction/1(x_I)
  Convert1/1 -> Phase Correction/2(x_Q)
  Register3/1 -> Divide1/1
  Register4/1 -> Divide/1
  Delay4/1 -> Delay12/1
  Delay12/1 -> Phase Correction/4(x_valid)
  Phase Correction/1(" x_I") -> " x_I"/1, Gateway Out7/1, Debug Outputs/1(I)
  Phase Correction/2(" x_Q") -> " x_Q"/1, Gateway Out2/1, Debug Outputs/2(Q)
  Phase Correction/3(" x_valid") -> " x_valid"/1, Gateway Out3/1, Debug Outputs/3(valid)
  Delay5/1 -> Delay14/1
  Delay14/1 -> Delay15/1
  Delay15/1 -> " x_ind"/1, Debug Outputs/4(x_ind)
  Delay9/1 -> Delay17/1
  Delay17/1 -> Delay18/1
  Delay18/1 -> " sym ind"/1
  Delay6/1 -> Phase Err Calc/3(x_valid), Delay4/1
  Gateway Out6/1 -> EQ/5  "Sym Ind"
  Gateway Out5/1 -> EQ/4  "X Ind"
  Gateway Out4/1 -> EQ/3  "X Valid"
  Gateway Out2/1 -> EQ/7  "EQ Q"
  Gateway Out1/1 -> EQ/2  "X_Q"
  Gateway Out/1 -> EQ/1  "X_I"
  Gateway Out7/1 -> EQ/6  "EQ I"
  Gateway Out3/1 -> EQ/8  "EQ Valid"
  Register5/1 -> Divide/2, Divide1/2

== system wlan_phy_rx_pmd/DSSS Rx/Demod ==
  Start : Inport
  I : Inport  Port=2
  Q : Inport  Port=3
  Bit-to-Byte : Subsystem
  DBPSK Demod : Subsystem
  DSSS Rx : Scope  NumInputPorts=7
      settings: TimeRange=56900  YMin=-1~-1~0.95~0~47.5~0~0  YMax=1~1~1.05~1~80~1~250
  De-spread : Subsystem
  Descramble : Subsystem
  Gateway Out{1..7} (7 alike) : xbsIndex_r4/Gateway Out  inherit_from_input=off  hdl_port=off  timing_constraint=None  locs_specified=off  LOCs={}  UseAsDAC=off  DACChannel='1'  block_type=gatewayout
  Header CRC : Subsystem
  Register8 : xbsIndex_r4/Register  init=0  rst=off  en=on  dbl_ovrd=off  block_type=register
  Sync : Subsystem
  Byte : Outport
  Byte Valid : Outport  Port=2
  Byte Ind : Outport  Port=3
  CRC Calc : Outport  Port=4
  tag scope: CS_DSSS_BIT_VALID=global
  wires:
  Q/1 -> De-spread/2(Q), Gateway Out3/1
  Start/1 -> De-spread/3(Sym Start), Gateway Out6/1
  I/1 -> De-spread/1(I), Gateway Out2/1
  De-spread/1(IQ Valid) -> DBPSK Demod/1(IQ Valid)
  De-spread/2(" I") -> DBPSK Demod/2(I)
  De-spread/3(" Q") -> DBPSK Demod/3(Q)
  DBPSK Demod/1(bit) -> Descramble/1(Bit)
  DBPSK Demod/2(bit valid) -> Descramble/2(Bit Valid)
  Descramble/1(" Bit") -> Register8/1, Sync/1(Bit)
  Descramble/2(" Bit Valid") -> Sync/2(Bit Valid), Register8/2, [CS_DSSS_BIT_VALID]
  Gateway Out2/1 -> DSSS Rx/1
  Gateway Out3/1 -> DSSS Rx/2
  Gateway Out6/1 -> DSSS Rx/3
  Gateway Out7/1 -> DSSS Rx/4
  Gateway Out1/1 -> DSSS Rx/5
  Gateway Out4/1 -> DSSS Rx/6
  Gateway Out5/1 -> DSSS Rx/7
  Sync/1(Payload Bit) -> Bit-to-Byte/1(Bit), Header CRC/1(Bit)
  Sync/2(" Bit Valid") -> Bit-to-Byte/2(Bit Valid), Header CRC/2(Bit Valid)
  Bit-to-Byte/3(Byte Ind) -> Byte Ind/1, Gateway Out1/1
  Bit-to-Byte/1(Byte) -> Byte/1, Gateway Out5/1
  Bit-to-Byte/2(Byte Valid) -> Byte Valid/1, Gateway Out4/1
  Register8/1 -> Gateway Out7/1
  Header CRC/1(CRC Calc) -> CRC Calc/1

== system wlan_phy_rx_pmd/DSSS Rx/Reset Gen ==
  note: Extend the DSSS reset event long enough to flush
      | the DSSS PHY pipeline and to reduce the
      | chance of spurious double-detections
  Reset : Inport
  Constant1 : xbsIndex_r4/Constant  const=127  gui_display_data_type=Fixed-point  arith_type=Unsigned  n_bits=7  bin_pt=0  preci_type=Single  exp_width=8  frac_width=24  explicit_period=off  period=1  equ=P=C  opselect=C  inp2=PCIN>>17  opr=+  inp1=P  carry=CIN  dbl_ovrd=off  block_type=constant
      settings: block_version=VER_STRING_GOES_HERE
  Counter1 : xbsIndex_r4/Counter  cnt_type=Free Running  cnt_to=Inf  operation=Up  start_count=0  cnt_by_val=1  arith_type=Unsigned  n_bits=7  bin_pt=0  load_pin=off  rst=on  en=on  explicit_period=off  period=1  dbl_ovrd=off  use_behavioral_HDL=off  implementation=Fabric  block_type=counter
  Down Sample3 : xbsIndex_r4/Down Sample  sample_ratio=8  sample_phase="Last Value of Frame  (most efficient)"  en=off  latency=1  dbl_ovrd=off  block_type=dsamp
  Inverter2 : xbsIndex_r4/Inverter  en=off  latency=0  dbl_ovrd=off  block_type=inv
  Logical1 : xbsIndex_r4/Logical  logical_function=OR  inputs=2  en=off  latency=0  precision=Full  arith_type=Unsigned  n_bits=8  bin_pt=2  align_bp=on  dbl_ovrd=off  block_type=logical
      settings: xl_area=[1 0 0 1 0 0 0]  block_version=VER_STRING_GOES_HERE
  Logical2 : xbsIndex_r4/Logical  logical_function=OR  inputs=2  en=off  latency=0  precision=Full  arith_type=Unsigned  n_bits=8  bin_pt=2  align_bp=on  dbl_ovrd=off  block_type=logical
      settings: xl_area=[1 0 0 1 0 0 0]  block_version=VER_STRING_GOES_HERE
  Posedge : Subsystem
  Relational1 : xbsIndex_r4/Relational  mode=a=b  en=off  latency=0  dbl_ovrd=off  block_type=relational
  S-R Latch : Subsystem
  S-R Latch2 : Subsystem
  negedge : Subsystem
  DSSS Rx Reset : Outport
  wires:
  Posedge/1(Q) -> S-R Latch/1(S), Logical1/1
  S-R Latch/1(Q) -> Logical2/2
  Counter1/1 -> Relational1/1
  Constant1/1 -> Relational1/2
  negedge/1(Q) -> S-R Latch2/1(S)
  S-R Latch2/1(Q) -> Counter1/2, Inverter2/1
  Relational1/1 -> S-R Latch2/2(R), S-R Latch/2(R)
  Inverter2/1 -> Logical1/2
  Reset/1 -> Posedge/1(D), negedge/1(D), Logical2/1
  Down Sample3/1 -> DSSS Rx Reset/1
  Logical1/1 -> Counter1/1
  Logical2/1 -> Down Sample3/1

== system wlan_phy_rx_pmd/DSSS Rx/S-R Latch1 ==
  S : Inport
  R : Inport  Port=2
  Constant1 : xbsIndex_r4/Constant  const=1  gui_display_data_type=Boolean  arith_type=Boolean  n_bits=1  bin_pt=0  preci_type=Single  exp_width=8  frac_width=24  explicit_period=off  period=1  equ=P=C  opselect=C  inp2=PCIN>>17  opr=+  inp1=P  carry=CIN  dbl_ovrd=off  block_type=constant
      settings: block_version=VER_STRING_GOES_HERE
  Convert1 : xbsIndex_r4/Convert  gui_display_data_type=Boolean  arith_type=Boolean  n_bits=1  bin_pt=0  float_type=Single  exp_bits=8  fraction_bits=24  quantization=Truncate  overflow=Wrap  en=off  latency=0  dbl_ovrd=off  pipeline=off  block_type=convert
  Convert2 : xbsIndex_r4/Convert  gui_display_data_type=Boolean  arith_type=Boolean  n_bits=1  bin_pt=0  float_type=Single  exp_bits=8  fraction_bits=24  quantization=Truncate  overflow=Wrap  en=off  latency=0  dbl_ovrd=off  pipeline=off  block_type=convert
  Register2 : xbsIndex_r4/Register  init=0  rst=on  en=on  dbl_ovrd=off  block_type=register
      settings: xl_area=[1 1 0 0 0 0 0]  block_version=VER_STRING_GOES_HERE
  Q : Outport
  wires:
  S/1 -> Convert2/1
  Register2/1 -> Q/1
  R/1 -> Convert1/1
  Constant1/1 -> Register2/1
  Convert1/1 -> Register2/2
  Convert2/1 -> Register2/3

== system wlan_phy_rx_pmd/DSSS Rx/SIGNAL Dec ==
  note: Convert to bytes, pad for FCS
  Byte : Inport
  Byte Valid : Inport  Port=2
  Byte Ind : Inport  Port=3
  CRC Calc : Inport  Port=4
  AddSub : xbsIndex_r4/AddSub  mode=Addition  use_carryin=off  use_carryout=off  en=off  latency=0  precision=User Defined  arith_type=Unsigned  n_bits=ceil(log2(MAX_NUM_BYTES))  bin_pt=0  quantization=Truncate  overflow=Wrap  dbl_ovrd=off  use_behavioral_HDL=off  hw_selection=Fabric  pipelined=off  block_type=addsub
  Concat : xbsIndex_r4/Concat  num_inputs=2  dbl_ovrd=off  block_type=concat
  Concat1 : xbsIndex_r4/Concat  num_inputs=2  dbl_ovrd=off  block_type=concat
  Constant1 : xbsIndex_r4/Constant  const=2  gui_display_data_type=Fixed-point  arith_type=Unsigned  n_bits=2  bin_pt=0  preci_type=Single  exp_width=8  frac_width=24  explicit_period=off  period=1  equ=P=C  opselect=C  inp2=PCIN>>17  opr=+  inp1=P  carry=CIN  dbl_ovrd=off  block_type=constant
  Constant2 : xbsIndex_r4/Constant  const=0  gui_display_data_type=Fixed-point  arith_type=Unsigned  n_bits=2  bin_pt=0  preci_type=Single  exp_width=8  frac_width=24  explicit_period=off  period=1  equ=P=C  opselect=C  inp2=PCIN>>17  opr=+  inp1=P  carry=CIN  dbl_ovrd=off  block_type=constant
  Constant3 : xbsIndex_r4/Constant  const=10  gui_display_data_type=Fixed-point  arith_type=Unsigned  n_bits=8  bin_pt=0  preci_type=Single  exp_width=8  frac_width=24  explicit_period=off  period=1  equ=P=C  opselect=C  inp2=PCIN>>17  opr=+  inp1=P  carry=CIN  dbl_ovrd=off  block_type=constant
  Constant4 : xbsIndex_r4/Constant  const=3  gui_display_data_type=Fixed-point  arith_type=Unsigned  n_bits=2  bin_pt=0  preci_type=Single  exp_width=8  frac_width=24  explicit_period=off  period=1  equ=P=C  opselect=C  inp2=PCIN>>17  opr=+  inp1=P  carry=CIN  dbl_ovrd=off  block_type=constant
  Constant6 : xbsIndex_r4/Constant  const=4  gui_display_data_type=Fixed-point  arith_type=Unsigned  n_bits=3  bin_pt=0  preci_type=Single  exp_width=8  frac_width=24  explicit_period=off  period=1  equ=P=C  opselect=C  inp2=PCIN>>17  opr=+  inp1=P  carry=CIN  dbl_ovrd=off  block_type=constant
  Constant7 : xbsIndex_r4/Constant  const=5  gui_display_data_type=Fixed-point  arith_type=Unsigned  n_bits=3  bin_pt=0  preci_type=Single  exp_width=8  frac_width=24  explicit_period=off  period=1  equ=P=C  opselect=C  inp2=PCIN>>17  opr=+  inp1=P  carry=CIN  dbl_ovrd=off  block_type=constant
  Constant9 : xbsIndex_r4/Constant  const=5  gui_display_data_type=Fixed-point  arith_type=Unsigned  n_bits=3  bin_pt=0  preci_type=Single  exp_width=8  frac_width=24  explicit_period=off  period=8  equ=P=C  opselect=C  inp2=PCIN>>17  opr=+  inp1=P  carry=CIN  dbl_ovrd=off  block_type=constant
  DOUT{,2,4..5} (4 alike) : xbsIndex_r4/Register  init=0  rst=on  en=on  dbl_ovrd=off  block_type=register
  DOUT3 : xbsIndex_r4/Register  init=4  rst=on  en=on  dbl_ovrd=off  block_type=register
  DSSS SIGNAL Decode : Scope  NumInputPorts=7
      settings: TimeRange=14500  YMin=0~0~972.8~0.95~-1~-1~-1  YMax=10~1~1075.2~1.05~1~1~1  SaveName=ScopeData44
  Delay1 : xbsIndex_r4/Delay  rst=off  infoeditControl=Selection of Reset will increase slice count due to use of real FFs and instead of SRLs  en=off  latency=1  dbl_ovrd=off  reg_retiming=off  block_type=delay
  Display : Display
  Display1 : Display
  Gateway Out{,1..7} (8 alike) : xbsIndex_r4/Gateway Out  inherit_from_input=off  hdl_port=off  timing_constraint=None  locs_specified=off  LOCs={}  UseAsDAC=off  DACChannel='1'  block_type=gatewayout
  Inverter1 : xbsIndex_r4/Inverter  en=off  latency=0  dbl_ovrd=off  block_type=inv
  Length Check : Subsystem
  Logical{,1..3,7..10} (8 alike) : xbsIndex_r4/Logical  logical_function=AND  inputs=2  en=off  latency=0  precision=Full  arith_type=Unsigned  n_bits=16  bin_pt=0  align_bp=on  dbl_ovrd=off  block_type=logical
  Logical6 : xbsIndex_r4/Logical  logical_function=OR  inputs=3  en=off  latency=0  precision=Full  arith_type=Unsigned  n_bits=16  bin_pt=0  align_bp=on  dbl_ovrd=off  block_type=logical
  Relational{1..2,4,6..7} (5 alike) : xbsIndex_r4/Relational  mode=a=b  en=off  latency=0  dbl_ovrd=off  block_type=relational
  Relational3 : xbsIndex_r4/Relational  mode=a!=b  en=off  latency=0  dbl_ovrd=off  block_type=relational
  Relational8 : xbsIndex_r4/Relational  mode=a!=b  en=off  latency=0  dbl_ovrd=off  block_type=relational
  Shift : xbsIndex_r4/Shift  shift_dir=Right  shift_bits=3  en=off  latency=0  precision=Full  arith_type="Signed  (2's comp)"  n_bits=16  bin_pt=14  quantization=Truncate  overflow=Wrap  dbl_ovrd=off  block_type=shift
  LENGTH : Outport
  Valid Header : Outport  Port=2
  Error : Outport  Port=3
  wires:
  Constant2/1 -> Relational2/2
  Relational1/1 -> Logical/2
  Relational2/1 -> Logical2/2
  Logical/1 -> DOUT2/3
  Logical2/1 -> DOUT/3
  Byte/1 -> DOUT/1, DOUT2/1, DOUT3/1, DOUT4/1, DOUT5/1, Gateway Out2/1
  [DSSS_RX_RESET] -> DOUT/2, DOUT2/2, DOUT3/2, DOUT4/2, DOUT5/2
  Byte Valid/1 -> Logical2/1, Logical/1, Logical3/1, Logical8/1, Logical7/1, Gateway Out3/1
  Constant1/1 -> Relational1/2
  Byte Ind/1 -> Relational1/1, Relational4/1, Relational7/1, Relational6/1, Relational2/1
  DOUT/1 -> Relational3/1, Gateway Out1/1
  Constant3/1 -> Relational3/2
  Relational4/1 -> Logical3/2
  Constant4/1 -> Relational4/2
  Logical3/1 -> DOUT3/3
  DOUT2/1 -> Concat/2
  DOUT3/1 -> Concat/1
  Concat/1 -> Gateway Out/1, Shift/1, Gateway Out6/1, Length Check/1(Len)
  Length Check/1(Invalid) -> Logical6/2
  Relational3/1 -> Logical6/1
  Gateway Out/1 -> Display/1
  Gateway Out1/1 -> Display1/1
  Constant6/1 -> Relational6/2
  Logical8/1 -> DOUT4/3, Delay1/1
  Relational6/1 -> Logical7/2
  Relational7/1 -> Logical8/2
  Constant7/1 -> Relational7/2
  DOUT4/1 -> Concat1/1
  DOUT5/1 -> Concat1/2
  Concat1/1 -> Relational8/1
  CRC Calc/1 -> Relational8/2
  Relational8/1 -> Logical9/1, Gateway Out7/1
  Delay1/1 -> Logical9/2, Logical10/2, Logical1/2
  Logical9/1 -> Logical6/3
  Logical7/1 -> DOUT5/3
  Constant9/1 -> AddSub/2
  AddSub/1 -> LENGTH/1
  Logical6/1 -> Inverter1/1, Logical1/1
  Inverter1/1 -> Logical10/1
  Logical10/1 -> Valid Header/1, Gateway Out4/1
  Logical1/1 -> Error/1, Gateway Out5/1
  Shift/1 -> AddSub/1
  Gateway Out2/1 -> DSSS SIGNAL Decode/1
  Gateway Out3/1 -> DSSS SIGNAL Decode/2
  Gateway Out4/1 -> DSSS SIGNAL Decode/6
  Gateway Out5/1 -> DSSS SIGNAL Decode/7
  Gateway Out6/1 -> DSSS SIGNAL Decode/3
  Gateway Out7/1 -> DSSS SIGNAL Decode/4
